simulink model "SeatClimaPreProcMgr"  (R2022b)
settings: Solver=FixedStepDiscrete  StartTime=0.0  StopTime=10.0  FixedStep=0.1  RelTol=1e-3
settings Model: Creator=blue.ge  LastModifiedBy=blue.ge
settings DataIOCC: SaveFormat=StructureWithTime
settings OptimizationCC: DefaultParameterBehavior=Inlined  EnhancedBackFolding=on  PassReuseOutputArgsAs=Individual arguments  ZeroExternalMemoryAtStartup=off  ZeroInternalMemoryAtStartup=off  OptimizeBlockOrder=speed  DifferentSizesBufferReuse=on  OptimizationCustomize=off  EfficientTunableParamExpr=on
settings DebuggingCC: ParameterTunabilityLossMsg=error
settings ModelReferenceCC: ModelReferenceNumInstancesAllowed=Single
settings SFSimCC: MATLABDynamicMemAlloc=off
settings RTWCC: Description=AUTOSAR  SystemTargetFile=autosar.tlc  GenCodeOnly=on  TemplateMakefile=ert_default_tmf
settings CodeAppCC: ForceParamTrailComments=on  IgnoreCustomStorageClasses=off  OperatorAnnotations=on  SimulinkDataObjDesc=on  SFDataObjDesc=on  InsertBlockDesc=on
settings STFCustomTargetCC: TargetLangStandard=C99 (ISO)  MultiwordLength=256  ParMdlRefBuildCompliant=on  CompOptLevelCompliant=on  IncludeMdlTerminateFcn=off  CombineOutputUpdateFcns=on  SuppressErrorStatus=on  ERTCustomFileBanners=on  SupportNonFinite=off  SupportComplex=off  SupportContinuousTime=off  SupportNonInlinedSFcns=off  RemoveResetFunc=on  MATLABClassNameForMDSCustomization=Simulink.SoftwareTarget.ERTCustomization  AutosarCompliant=on  UseToolchainInfoCompliant=on  LookupTableObjectStructAxisOrder=2,1,3,4,...  LUTObjectStructOrderEvenSpacing=Size,Table,Breakpoints  SystemTargetFile=autosar.tlc
settings ERTTargetCC: TargetLangStandard=C99 (ISO)  ConcurrentExecutionCompliant=off  IncludeMdlTerminateFcn=off  SuppressErrorStatus=on  SupportNonFinite=off  SupportComplex=off  ModelStepFunctionPrototypeControlCompliant=off  CPPClassGenCompliant=off  AutosarCompliant=on  LookupTableObjectStructAxisOrder=2,1,3,4,...  LUTObjectStructOrderEvenSpacing=Size,Table,Breakpoints  InlinedParameterPlacement=NonHierarchical  ERTCustomFileTemplate=example_file_process.tlc

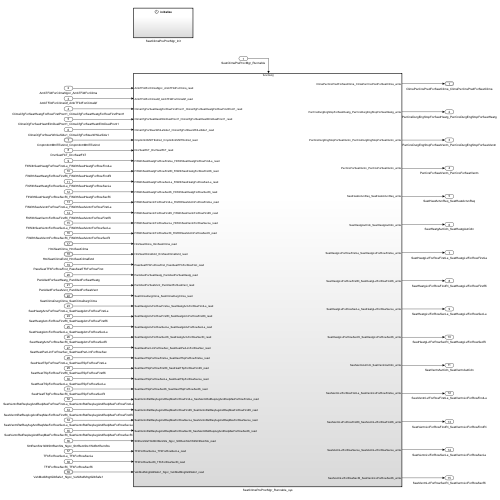
[thumbnail: Simulink's stored preview of the top-level diagram]
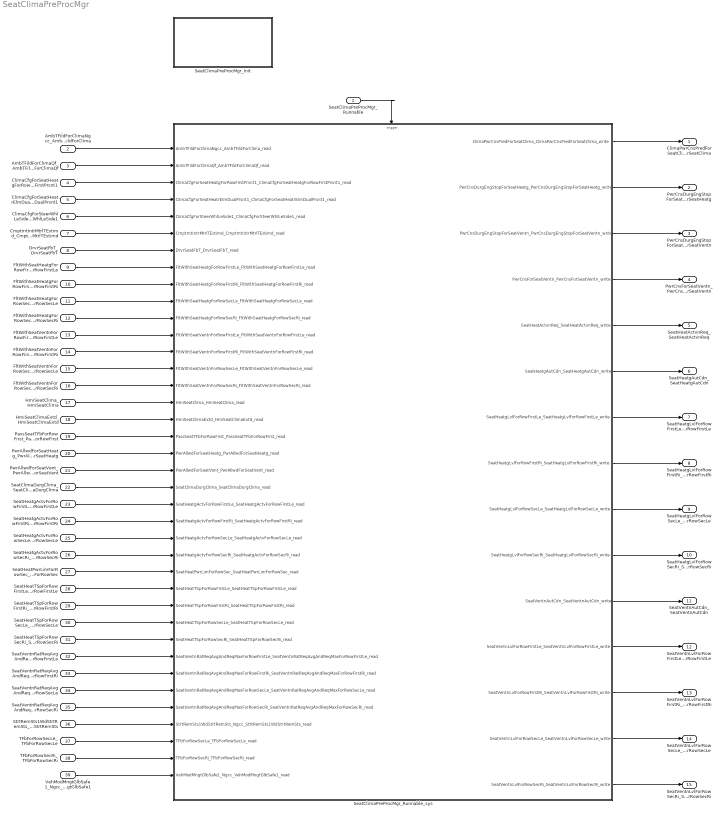
== system SeatClimaPreProcMgr ==
  SeatClimaPreProcMgr_Runnable : Inport  SampleTime=0.1  OutputFunctionCall=on
  AmbTFildForClimaNgcc_AmbTFildForClima : Inport  Port=2  OutDataTypeStr=T30  PortDimensions=1
  AmbTFildForClimaQf_AmbTFildForClimaQf : Inport  Port=3  OutDataTypeStr=Enum: GenQf1  PortDimensions=1
  ClimaCfgForSeatHeatgForRowFirstPrsnt1_ClimaCfgForSeatHeatgForRowFirstPrsnt1 : Inport  Port=4  OutDataTypeStr=Boolean  PortDimensions=1
  ClimaCfgForSeatHeatrElmDualPrsnt1_ClimaCfgForSeatHeatrElmDualPrsnt1 : Inport  Port=5  OutDataTypeStr=Boolean  PortDimensions=1
  ClimaCfgForSteerWhlLeSide1_ClimaCfgForSteerWhlLeSide1 : Inport  Port=6  OutDataTypeStr=Boolean  PortDimensions=1
  CmptmtIntrMtrlTEstimd_CmptmtIntrMtrlTEstimd : Inport  Port=7  OutDataTypeStr=Bus: MtrlSnsrT  PortDimensions=1  BusOutputAsStruct=on
  DrvrSeatFbT_DrvrSeatFbT : Inport  Port=8  OutDataTypeStr=T30  PortDimensions=1
  FltWithSeatHeatgForRowFirstLe_FltWithSeatHeatgForRowFirstLe : Inport  Port=9  OutDataTypeStr=Boolean  PortDimensions=1
  FltWithSeatHeatgForRowFirstRi_FltWithSeatHeatgForRowFirstRi : Inport  Port=10  OutDataTypeStr=Boolean  PortDimensions=1
  FltWithSeatHeatgForRowSecLe_FltWithSeatHeatgForRowSecLe : Inport  Port=11  OutDataTypeStr=Boolean  PortDimensions=1
  FltWithSeatHeatgForRowSecRi_FltWithSeatHeatgForRowSecRi : Inport  Port=12  OutDataTypeStr=Boolean  PortDimensions=1
  FltWithSeatVentnForRowFirstLe_FltWithSeatVentnForRowFirstLe : Inport  Port=13  OutDataTypeStr=Boolean  PortDimensions=1
  FltWithSeatVentnForRowFirstRi_FltWithSeatVentnForRowFirstRi : Inport  Port=14  OutDataTypeStr=Boolean  PortDimensions=1
  FltWithSeatVentnForRowSecLe_FltWithSeatVentnForRowSecLe : Inport  Port=15  OutDataTypeStr=Boolean  PortDimensions=1
  FltWithSeatVentnForRowSecRi_FltWithSeatVentnForRowSecRi : Inport  Port=16  OutDataTypeStr=Boolean  PortDimensions=1
  HmiSeatClima_HmiSeatClima : Inport  Port=17  OutDataTypeStr=Bus: HmiSeatClima  PortDimensions=1  BusOutputAsStruct=on
  HmiSeatClimaExtd_HmiSeatClimaExtd : Inport  Port=18  OutDataTypeStr=Bus: HmiSeatClimaExtd  PortDimensions=1  BusOutputAsStruct=on
  PassSeatTFbForRowFirst_PassSeatTFbForRowFirst : Inport  Port=19  OutDataTypeStr=T30  PortDimensions=1
  PwrAllwdForSeatHeatg_PwrAllwdForSeatHeatg : Inport  Port=20  OutDataTypeStr=Pwr33  PortDimensions=1
  PwrAllwdForSeatVent_PwrAllwdForSeatVent : Inport  Port=21  OutDataTypeStr=Pwr33  PortDimensions=1
  SeatClimaDurgClima_SeatClimaDurgClima : Inport  Port=22  OutDataTypeStr=Bus: HmiSeatClima  PortDimensions=1  BusOutputAsStruct=on
  SeatHeatgActvForRowFirstLe_SeatHeatgActvForRowFirstLe : Inport  Port=23  OutDataTypeStr=Boolean  PortDimensions=1
  SeatHeatgActvForRowFirstRi_SeatHeatgActvForRowFirstRi : Inport  Port=24  OutDataTypeStr=Boolean  PortDimensions=1
  SeatHeatgActvForRowSecLe_SeatHeatgActvForRowSecLe : Inport  Port=25  OutDataTypeStr=Boolean  PortDimensions=1
  SeatHeatgActvForRowSecRi_SeatHeatgActvForRowSecRi : Inport  Port=26  OutDataTypeStr=Boolean  PortDimensions=1
  SeatHeatPwrLimForRowSec_SeatHeatPwrLimForRowSec : Inport  Port=27  OutDataTypeStr=Perc30  PortDimensions=1
  SeatHeatTSpForRowFirstLe_SeatHeatTSpForRowFirstLe : Inport  Port=28  OutDataTypeStr=T30  PortDimensions=1
  SeatHeatTSpForRowFirstRi_SeatHeatTSpForRowFirstRi : Inport  Port=29  OutDataTypeStr=T30  PortDimensions=1
  SeatHeatTSpForRowSecLe_SeatHeatTSpForRowSecLe : Inport  Port=30  OutDataTypeStr=T30  PortDimensions=1
  SeatHeatTSpForRowSecRi_SeatHeatTSpForRowSecRi : Inport  Port=31  OutDataTypeStr=T30  PortDimensions=1
  SeatVentnRatReqAvgAndReqMaxForRowFirstLe_SeatVentnRatReqAvgAndReqMaxForRowFirstLe : Inport  Port=32  OutDataTypeStr=Fac30  PortDimensions=1
  SeatVentnRatReqAvgAndReqMaxForRowFirstRi_SeatVentnRatReqAvgAndReqMaxForRowFirstRi : Inport  Port=33  OutDataTypeStr=Fac30  PortDimensions=1
  SeatVentnRatReqAvgAndReqMaxForRowSecLe_SeatVentnRatReqAvgAndReqMaxForRowSecLe : Inport  Port=34  OutDataTypeStr=Fac30  PortDimensions=1
  SeatVentnRatReqAvgAndReqMaxForRowSecRi_SeatVentnRatReqAvgAndReqMaxForRowSecRi : Inport  Port=35  OutDataTypeStr=Fac30  PortDimensions=1
  StrtRemSts1WdStrtRemSts_Ngcc_StrtRemSts1WdStrtRemSts : Inport  Port=36  OutDataTypeStr=Enum: StrtRemSts1  PortDimensions=1
  TFbForRowSecLe_TFbForRowSecLe : Inport  Port=37  OutDataTypeStr=T30  PortDimensions=1
  TFbForRowSecRi_TFbForRowSecRi : Inport  Port=38  OutDataTypeStr=T30  PortDimensions=1
  VehModMngtGlbSafe1_Ngcc_VehModMngtGlbSafe1 : Inport  Port=39  OutDataTypeStr=Bus: VehModMngtGlbSafe1  PortDimensions=1  BusOutputAsStruct=on
  SeatClimaPreProcMgr_Init : Subsystem
  SeatClimaPreProcMgr_Runnable_sys : Subsystem
  ClimaPwrCnsPredForSeatClima_ClimaPwrCnsPredForSeatClima : Outport  OutDataTypeStr=Bus: ClimaPwrCnsPredRec1  PortDimensions=1  BusOutputAsStruct=on
  PwrCnsDurgEngStopForSeatHeatg_PwrCnsDurgEngStopForSeatHeatg : Outport  Port=2  OutDataTypeStr=Pwr33  PortDimensions=1
  PwrCnsDurgEngStopForSeatVentn_PwrCnsDurgEngStopForSeatVentn : Outport  Port=3  OutDataTypeStr=Pwr33  PortDimensions=1
  PwrCnsForSeatVentn_PwrCnsForSeatVentn : Outport  Port=4  OutDataTypeStr=Pwr33  PortDimensions=1
  SeatHeatActvnReq_SeatHeatActvnReq : Outport  Port=5  OutDataTypeStr=Enum: OnOff1  PortDimensions=1
  SeatHeatgAutCdn_SeatHeatgAutCdn : Outport  Port=6  OutDataTypeStr=Enum: Flg1  PortDimensions=1
  SeatHeatgLvlForRowFirstLe_SeatHeatgLvlForRowFirstLe : Outport  Port=7  OutDataTypeStr=Enum: SeatClimaLvl  PortDimensions=1
  SeatHeatgLvlForRowFirstRi_SeatHeatgLvlForRowFirstRi : Outport  Port=8  OutDataTypeStr=Enum: SeatClimaLvl  PortDimensions=1
  SeatHeatgLvlForRowSecLe_SeatHeatgLvlForRowSecLe : Outport  Port=9  OutDataTypeStr=Enum: SeatClimaLvl  PortDimensions=1
  SeatHeatgLvlForRowSecRi_SeatHeatgLvlForRowSecRi : Outport  Port=10  OutDataTypeStr=Enum: SeatClimaLvl  PortDimensions=1
  SeatVentnAutCdn_SeatVentnAutCdn : Outport  Port=11  OutDataTypeStr=Enum: Flg1  PortDimensions=1
  SeatVentnLvlForRowFirstLe_SeatVentnLvlForRowFirstLe : Outport  Port=12  OutDataTypeStr=Enum: SeatClimaLvl  PortDimensions=1
  SeatVentnLvlForRowFirstRi_SeatVentnLvlForRowFirstRi : Outport  Port=13  OutDataTypeStr=Enum: SeatClimaLvl  PortDimensions=1
  SeatVentnLvlForRowSecLe_SeatVentnLvlForRowSecLe : Outport  Port=14  OutDataTypeStr=Enum: SeatClimaLvl  PortDimensions=1
  SeatVentnLvlForRowSecRi_SeatVentnLvlForRowSecRi : Outport  Port=15  OutDataTypeStr=Enum: SeatClimaLvl  PortDimensions=1
  wires:
  AmbTFildForClimaNgcc_AmbTFildForClima/1 -> SeatClimaPreProcMgr_Runnable_sys/1(AmbTFildForClimaNgcc_AmbTFildForClima_read)
  AmbTFildForClimaQf_AmbTFildForClimaQf/1 -> SeatClimaPreProcMgr_Runnable_sys/2(AmbTFildForClimaQf_AmbTFildForClimaQf_read)
  ClimaCfgForSeatHeatgForRowFirstPrsnt1_ClimaCfgForSeatHeatgForRowFirstPrsnt1/1 -> SeatClimaPreProcMgr_Runnable_sys/3(ClimaCfgForSeatHeatgForRowFirstPrsnt1_ClimaCfgForSeatHeatgForRowFirstPrsnt1_read)
  ClimaCfgForSeatHeatrElmDualPrsnt1_ClimaCfgForSeatHeatrElmDualPrsnt1/1 -> SeatClimaPreProcMgr_Runnable_sys/4(ClimaCfgForSeatHeatrElmDualPrsnt1_ClimaCfgForSeatHeatrElmDualPrsnt1_read)
  ClimaCfgForSteerWhlLeSide1_ClimaCfgForSteerWhlLeSide1/1 -> SeatClimaPreProcMgr_Runnable_sys/5(ClimaCfgForSteerWhlLeSide1_ClimaCfgForSteerWhlLeSide1_read)
  CmptmtIntrMtrlTEstimd_CmptmtIntrMtrlTEstimd/1 -> SeatClimaPreProcMgr_Runnable_sys/6(CmptmtIntrMtrlTEstimd_CmptmtIntrMtrlTEstimd_read)
  DrvrSeatFbT_DrvrSeatFbT/1 -> SeatClimaPreProcMgr_Runnable_sys/7(DrvrSeatFbT_DrvrSeatFbT_read)
  FltWithSeatHeatgForRowFirstLe_FltWithSeatHeatgForRowFirstLe/1 -> SeatClimaPreProcMgr_Runnable_sys/8(FltWithSeatHeatgForRowFirstLe_FltWithSeatHeatgForRowFirstLe_read)
  FltWithSeatHeatgForRowFirstRi_FltWithSeatHeatgForRowFirstRi/1 -> SeatClimaPreProcMgr_Runnable_sys/9(FltWithSeatHeatgForRowFirstRi_FltWithSeatHeatgForRowFirstRi_read)
  FltWithSeatHeatgForRowSecLe_FltWithSeatHeatgForRowSecLe/1 -> SeatClimaPreProcMgr_Runnable_sys/10(FltWithSeatHeatgForRowSecLe_FltWithSeatHeatgForRowSecLe_read)
  FltWithSeatHeatgForRowSecRi_FltWithSeatHeatgForRowSecRi/1 -> SeatClimaPreProcMgr_Runnable_sys/11(FltWithSeatHeatgForRowSecRi_FltWithSeatHeatgForRowSecRi_read)
  FltWithSeatVentnForRowFirstLe_FltWithSeatVentnForRowFirstLe/1 -> SeatClimaPreProcMgr_Runnable_sys/12(FltWithSeatVentnForRowFirstLe_FltWithSeatVentnForRowFirstLe_read)
  FltWithSeatVentnForRowFirstRi_FltWithSeatVentnForRowFirstRi/1 -> SeatClimaPreProcMgr_Runnable_sys/13(FltWithSeatVentnForRowFirstRi_FltWithSeatVentnForRowFirstRi_read)
  FltWithSeatVentnForRowSecLe_FltWithSeatVentnForRowSecLe/1 -> SeatClimaPreProcMgr_Runnable_sys/14(FltWithSeatVentnForRowSecLe_FltWithSeatVentnForRowSecLe_read)
  FltWithSeatVentnForRowSecRi_FltWithSeatVentnForRowSecRi/1 -> SeatClimaPreProcMgr_Runnable_sys/15(FltWithSeatVentnForRowSecRi_FltWithSeatVentnForRowSecRi_read)
  HmiSeatClima_HmiSeatClima/1 -> SeatClimaPreProcMgr_Runnable_sys/16(HmiSeatClima_HmiSeatClima_read)
  HmiSeatClimaExtd_HmiSeatClimaExtd/1 -> SeatClimaPreProcMgr_Runnable_sys/17(HmiSeatClimaExtd_HmiSeatClimaExtd_read)
  PassSeatTFbForRowFirst_PassSeatTFbForRowFirst/1 -> SeatClimaPreProcMgr_Runnable_sys/18(PassSeatTFbForRowFirst_PassSeatTFbForRowFirst_read)
  PwrAllwdForSeatHeatg_PwrAllwdForSeatHeatg/1 -> SeatClimaPreProcMgr_Runnable_sys/19(PwrAllwdForSeatHeatg_PwrAllwdForSeatHeatg_read)
  PwrAllwdForSeatVent_PwrAllwdForSeatVent/1 -> SeatClimaPreProcMgr_Runnable_sys/20(PwrAllwdForSeatVent_PwrAllwdForSeatVent_read)
  SeatClimaDurgClima_SeatClimaDurgClima/1 -> SeatClimaPreProcMgr_Runnable_sys/21(SeatClimaDurgClima_SeatClimaDurgClima_read)
  SeatHeatgActvForRowFirstLe_SeatHeatgActvForRowFirstLe/1 -> SeatClimaPreProcMgr_Runnable_sys/22(SeatHeatgActvForRowFirstLe_SeatHeatgActvForRowFirstLe_read)
  SeatHeatgActvForRowFirstRi_SeatHeatgActvForRowFirstRi/1 -> SeatClimaPreProcMgr_Runnable_sys/23(SeatHeatgActvForRowFirstRi_SeatHeatgActvForRowFirstRi_read)
  SeatHeatgActvForRowSecLe_SeatHeatgActvForRowSecLe/1 -> SeatClimaPreProcMgr_Runnable_sys/24(SeatHeatgActvForRowSecLe_SeatHeatgActvForRowSecLe_read)
  SeatHeatgActvForRowSecRi_SeatHeatgActvForRowSecRi/1 -> SeatClimaPreProcMgr_Runnable_sys/25(SeatHeatgActvForRowSecRi_SeatHeatgActvForRowSecRi_read)
  SeatHeatPwrLimForRowSec_SeatHeatPwrLimForRowSec/1 -> SeatClimaPreProcMgr_Runnable_sys/26(SeatHeatPwrLimForRowSec_SeatHeatPwrLimForRowSec_read)
  SeatHeatTSpForRowFirstLe_SeatHeatTSpForRowFirstLe/1 -> SeatClimaPreProcMgr_Runnable_sys/27(SeatHeatTSpForRowFirstLe_SeatHeatTSpForRowFirstLe_read)
  SeatHeatTSpForRowFirstRi_SeatHeatTSpForRowFirstRi/1 -> SeatClimaPreProcMgr_Runnable_sys/28(SeatHeatTSpForRowFirstRi_SeatHeatTSpForRowFirstRi_read)
  SeatHeatTSpForRowSecLe_SeatHeatTSpForRowSecLe/1 -> SeatClimaPreProcMgr_Runnable_sys/29(SeatHeatTSpForRowSecLe_SeatHeatTSpForRowSecLe_read)
  SeatHeatTSpForRowSecRi_SeatHeatTSpForRowSecRi/1 -> SeatClimaPreProcMgr_Runnable_sys/30(SeatHeatTSpForRowSecRi_SeatHeatTSpForRowSecRi_read)
  SeatVentnRatReqAvgAndReqMaxForRowFirstLe_SeatVentnRatReqAvgAndReqMaxForRowFirstLe/1 -> SeatClimaPreProcMgr_Runnable_sys/31(SeatVentnRatReqAvgAndReqMaxForRowFirstLe_SeatVentnRatReqAvgAndReqMaxForRowFirstLe_read)
  SeatVentnRatReqAvgAndReqMaxForRowFirstRi_SeatVentnRatReqAvgAndReqMaxForRowFirstRi/1 -> SeatClimaPreProcMgr_Runnable_sys/32(SeatVentnRatReqAvgAndReqMaxForRowFirstRi_SeatVentnRatReqAvgAndReqMaxForRowFirstRi_read)
  SeatVentnRatReqAvgAndReqMaxForRowSecLe_SeatVentnRatReqAvgAndReqMaxForRowSecLe/1 -> SeatClimaPreProcMgr_Runnable_sys/33(SeatVentnRatReqAvgAndReqMaxForRowSecLe_SeatVentnRatReqAvgAndReqMaxForRowSecLe_read)
  SeatVentnRatReqAvgAndReqMaxForRowSecRi_SeatVentnRatReqAvgAndReqMaxForRowSecRi/1 -> SeatClimaPreProcMgr_Runnable_sys/34(SeatVentnRatReqAvgAndReqMaxForRowSecRi_SeatVentnRatReqAvgAndReqMaxForRowSecRi_read)
  StrtRemSts1WdStrtRemSts_Ngcc_StrtRemSts1WdStrtRemSts/1 -> SeatClimaPreProcMgr_Runnable_sys/35(StrtRemSts1WdStrtRemSts_Ngcc_StrtRemSts1WdStrtRemSts_read)
  TFbForRowSecLe_TFbForRowSecLe/1 -> SeatClimaPreProcMgr_Runnable_sys/36(TFbForRowSecLe_TFbForRowSecLe_read)
  TFbForRowSecRi_TFbForRowSecRi/1 -> SeatClimaPreProcMgr_Runnable_sys/37(TFbForRowSecRi_TFbForRowSecRi_read)
  VehModMngtGlbSafe1_Ngcc_VehModMngtGlbSafe1/1 -> SeatClimaPreProcMgr_Runnable_sys/38(VehModMngtGlbSafe1_Ngcc_VehModMngtGlbSafe1_read)
  SeatClimaPreProcMgr_Runnable_sys/1(ClimaPwrCnsPredForSeatClima_ClimaPwrCnsPredForSeatClima_write) -> ClimaPwrCnsPredForSeatClima_ClimaPwrCnsPredForSeatClima/1
  SeatClimaPreProcMgr_Runnable_sys/2(PwrCnsDurgEngStopForSeatHeatg_PwrCnsDurgEngStopForSeatHeatg_write) -> PwrCnsDurgEngStopForSeatHeatg_PwrCnsDurgEngStopForSeatHeatg/1
  SeatClimaPreProcMgr_Runnable_sys/3(PwrCnsDurgEngStopForSeatVentn_PwrCnsDurgEngStopForSeatVentn_write) -> PwrCnsDurgEngStopForSeatVentn_PwrCnsDurgEngStopForSeatVentn/1
  SeatClimaPreProcMgr_Runnable_sys/4(PwrCnsForSeatVentn_PwrCnsForSeatVentn_write) -> PwrCnsForSeatVentn_PwrCnsForSeatVentn/1
  SeatClimaPreProcMgr_Runnable_sys/5(SeatHeatActvnReq_SeatHeatActvnReq_write) -> SeatHeatActvnReq_SeatHeatActvnReq/1
  SeatClimaPreProcMgr_Runnable_sys/6(SeatHeatgAutCdn_SeatHeatgAutCdn_write) -> SeatHeatgAutCdn_SeatHeatgAutCdn/1
  SeatClimaPreProcMgr_Runnable_sys/7(SeatHeatgLvlForRowFirstLe_SeatHeatgLvlForRowFirstLe_write) -> SeatHeatgLvlForRowFirstLe_SeatHeatgLvlForRowFirstLe/1
  SeatClimaPreProcMgr_Runnable_sys/8(SeatHeatgLvlForRowFirstRi_SeatHeatgLvlForRowFirstRi_write) -> SeatHeatgLvlForRowFirstRi_SeatHeatgLvlForRowFirstRi/1
  SeatClimaPreProcMgr_Runnable_sys/9(SeatHeatgLvlForRowSecLe_SeatHeatgLvlForRowSecLe_write) -> SeatHeatgLvlForRowSecLe_SeatHeatgLvlForRowSecLe/1
  SeatClimaPreProcMgr_Runnable_sys/10(SeatHeatgLvlForRowSecRi_SeatHeatgLvlForRowSecRi_write) -> SeatHeatgLvlForRowSecRi_SeatHeatgLvlForRowSecRi/1
  SeatClimaPreProcMgr_Runnable_sys/11(SeatVentnAutCdn_SeatVentnAutCdn_write) -> SeatVentnAutCdn_SeatVentnAutCdn/1
  SeatClimaPreProcMgr_Runnable_sys/12(SeatVentnLvlForRowFirstLe_SeatVentnLvlForRowFirstLe_write) -> SeatVentnLvlForRowFirstLe_SeatVentnLvlForRowFirstLe/1
  SeatClimaPreProcMgr_Runnable_sys/13(SeatVentnLvlForRowFirstRi_SeatVentnLvlForRowFirstRi_write) -> SeatVentnLvlForRowFirstRi_SeatVentnLvlForRowFirstRi/1
  SeatClimaPreProcMgr_Runnable_sys/14(SeatVentnLvlForRowSecLe_SeatVentnLvlForRowSecLe_write) -> SeatVentnLvlForRowSecLe_SeatVentnLvlForRowSecLe/1
  SeatClimaPreProcMgr_Runnable_sys/15(SeatVentnLvlForRowSecRi_SeatVentnLvlForRowSecRi_write) -> SeatVentnLvlForRowSecRi_SeatVentnLvlForRowSecRi/1
  SeatClimaPreProcMgr_Runnable/1 -> SeatClimaPreProcMgr_Runnable_sys/Trigger

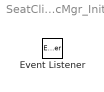
== system SeatClimaPreProcMgr/SeatClimaPreProcMgr_Init ==
  Event Listener : EventListener  EventName=reset

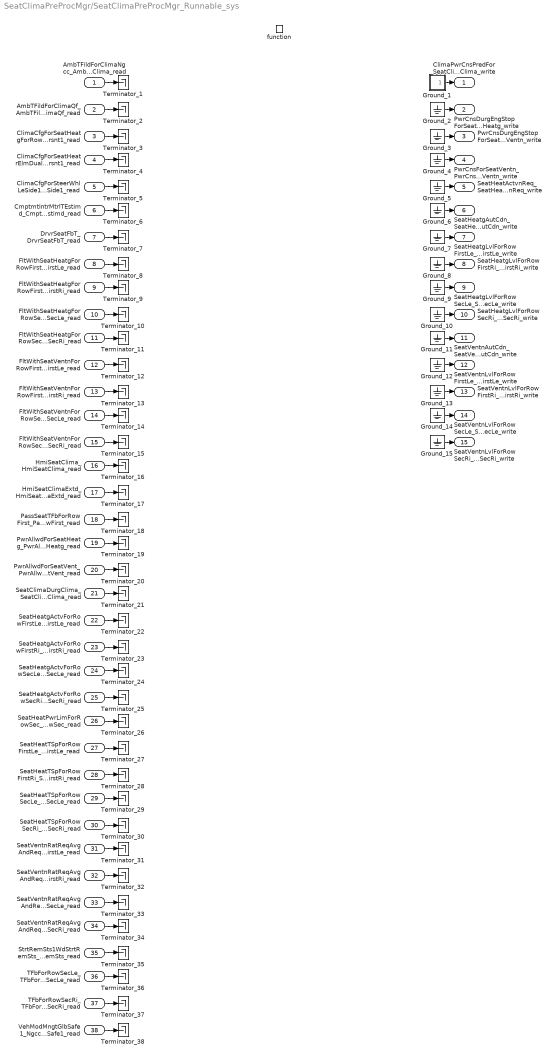
== system SeatClimaPreProcMgr/SeatClimaPreProcMgr_Runnable_sys ==
  AmbTFildForClimaNgcc_AmbTFildForClima_read : Inport
  AmbTFildForClimaQf_AmbTFildForClimaQf_read : Inport  Port=2
  ClimaCfgForSeatHeatgForRowFirstPrsnt1_ClimaCfgForSeatHeatgForRowFirstPrsnt1_read : Inport  Port=3
  ClimaCfgForSeatHeatrElmDualPrsnt1_ClimaCfgForSeatHeatrElmDualPrsnt1_read : Inport  Port=4
  ClimaCfgForSteerWhlLeSide1_ClimaCfgForSteerWhlLeSide1_read : Inport  Port=5
  CmptmtIntrMtrlTEstimd_CmptmtIntrMtrlTEstimd_read : Inport  Port=6
  DrvrSeatFbT_DrvrSeatFbT_read : Inport  Port=7
  FltWithSeatHeatgForRowFirstLe_FltWithSeatHeatgForRowFirstLe_read : Inport  Port=8
  FltWithSeatHeatgForRowFirstRi_FltWithSeatHeatgForRowFirstRi_read : Inport  Port=9
  FltWithSeatHeatgForRowSecLe_FltWithSeatHeatgForRowSecLe_read : Inport  Port=10
  FltWithSeatHeatgForRowSecRi_FltWithSeatHeatgForRowSecRi_read : Inport  Port=11
  FltWithSeatVentnForRowFirstLe_FltWithSeatVentnForRowFirstLe_read : Inport  Port=12
  FltWithSeatVentnForRowFirstRi_FltWithSeatVentnForRowFirstRi_read : Inport  Port=13
  FltWithSeatVentnForRowSecLe_FltWithSeatVentnForRowSecLe_read : Inport  Port=14
  FltWithSeatVentnForRowSecRi_FltWithSeatVentnForRowSecRi_read : Inport  Port=15
  HmiSeatClima_HmiSeatClima_read : Inport  Port=16
  HmiSeatClimaExtd_HmiSeatClimaExtd_read : Inport  Port=17
  PassSeatTFbForRowFirst_PassSeatTFbForRowFirst_read : Inport  Port=18
  PwrAllwdForSeatHeatg_PwrAllwdForSeatHeatg_read : Inport  Port=19
  PwrAllwdForSeatVent_PwrAllwdForSeatVent_read : Inport  Port=20
  SeatClimaDurgClima_SeatClimaDurgClima_read : Inport  Port=21
  SeatHeatgActvForRowFirstLe_SeatHeatgActvForRowFirstLe_read : Inport  Port=22
  SeatHeatgActvForRowFirstRi_SeatHeatgActvForRowFirstRi_read : Inport  Port=23
  SeatHeatgActvForRowSecLe_SeatHeatgActvForRowSecLe_read : Inport  Port=24
  SeatHeatgActvForRowSecRi_SeatHeatgActvForRowSecRi_read : Inport  Port=25
  SeatHeatPwrLimForRowSec_SeatHeatPwrLimForRowSec_read : Inport  Port=26
  SeatHeatTSpForRowFirstLe_SeatHeatTSpForRowFirstLe_read : Inport  Port=27
  SeatHeatTSpForRowFirstRi_SeatHeatTSpForRowFirstRi_read : Inport  Port=28
  SeatHeatTSpForRowSecLe_SeatHeatTSpForRowSecLe_read : Inport  Port=29
  SeatHeatTSpForRowSecRi_SeatHeatTSpForRowSecRi_read : Inport  Port=30
  SeatVentnRatReqAvgAndReqMaxForRowFirstLe_SeatVentnRatReqAvgAndReqMaxForRowFirstLe_read : Inport  Port=31
  SeatVentnRatReqAvgAndReqMaxForRowFirstRi_SeatVentnRatReqAvgAndReqMaxForRowFirstRi_read : Inport  Port=32
  SeatVentnRatReqAvgAndReqMaxForRowSecLe_SeatVentnRatReqAvgAndReqMaxForRowSecLe_read : Inport  Port=33
  SeatVentnRatReqAvgAndReqMaxForRowSecRi_SeatVentnRatReqAvgAndReqMaxForRowSecRi_read : Inport  Port=34
  StrtRemSts1WdStrtRemSts_Ngcc_StrtRemSts1WdStrtRemSts_read : Inport  Port=35
  TFbForRowSecLe_TFbForRowSecLe_read : Inport  Port=36
  TFbForRowSecRi_TFbForRowSecRi_read : Inport  Port=37
  VehModMngtGlbSafe1_Ngcc_VehModMngtGlbSafe1_read : Inport  Port=38
  function : TriggerPort  TriggerType=function-call  VariantControl=(inherit)  SampleTimeType=periodic  SampleTime=-1
  Ground_1 : Subsystem
  Ground_{2..15} (14 alike) : Ground
  Terminator_{1..38} (38 alike) : Terminator
  ClimaPwrCnsPredForSeatClima_ClimaPwrCnsPredForSeatClima_write : Outport
      settings: EnsureOutportIsVirtual=on
  PwrCnsDurgEngStopForSeatHeatg_PwrCnsDurgEngStopForSeatHeatg_write : Outport  Port=2
      settings: EnsureOutportIsVirtual=on
  PwrCnsDurgEngStopForSeatVentn_PwrCnsDurgEngStopForSeatVentn_write : Outport  Port=3
      settings: EnsureOutportIsVirtual=on
  PwrCnsForSeatVentn_PwrCnsForSeatVentn_write : Outport  Port=4
      settings: EnsureOutportIsVirtual=on
  SeatHeatActvnReq_SeatHeatActvnReq_write : Outport  Port=5
      settings: EnsureOutportIsVirtual=on
  SeatHeatgAutCdn_SeatHeatgAutCdn_write : Outport  Port=6
      settings: EnsureOutportIsVirtual=on
  SeatHeatgLvlForRowFirstLe_SeatHeatgLvlForRowFirstLe_write : Outport  Port=7
      settings: EnsureOutportIsVirtual=on
  SeatHeatgLvlForRowFirstRi_SeatHeatgLvlForRowFirstRi_write : Outport  Port=8
      settings: EnsureOutportIsVirtual=on
  SeatHeatgLvlForRowSecLe_SeatHeatgLvlForRowSecLe_write : Outport  Port=9
      settings: EnsureOutportIsVirtual=on
  SeatHeatgLvlForRowSecRi_SeatHeatgLvlForRowSecRi_write : Outport  Port=10
      settings: EnsureOutportIsVirtual=on
  SeatVentnAutCdn_SeatVentnAutCdn_write : Outport  Port=11
      settings: EnsureOutportIsVirtual=on
  SeatVentnLvlForRowFirstLe_SeatVentnLvlForRowFirstLe_write : Outport  Port=12
      settings: EnsureOutportIsVirtual=on
  SeatVentnLvlForRowFirstRi_SeatVentnLvlForRowFirstRi_write : Outport  Port=13
      settings: EnsureOutportIsVirtual=on
  SeatVentnLvlForRowSecLe_SeatVentnLvlForRowSecLe_write : Outport  Port=14
      settings: EnsureOutportIsVirtual=on
  SeatVentnLvlForRowSecRi_SeatVentnLvlForRowSecRi_write : Outport  Port=15
      settings: EnsureOutportIsVirtual=on
  wires:
  AmbTFildForClimaNgcc_AmbTFildForClima_read/1 -> Terminator_1/1
  AmbTFildForClimaQf_AmbTFildForClimaQf_read/1 -> Terminator_2/1
  ClimaCfgForSeatHeatgForRowFirstPrsnt1_ClimaCfgForSeatHeatgForRowFirstPrsnt1_read/1 -> Terminator_3/1
  ClimaCfgForSeatHeatrElmDualPrsnt1_ClimaCfgForSeatHeatrElmDualPrsnt1_read/1 -> Terminator_4/1
  ClimaCfgForSteerWhlLeSide1_ClimaCfgForSteerWhlLeSide1_read/1 -> Terminator_5/1
  CmptmtIntrMtrlTEstimd_CmptmtIntrMtrlTEstimd_read/1 -> Terminator_6/1
  DrvrSeatFbT_DrvrSeatFbT_read/1 -> Terminator_7/1
  FltWithSeatHeatgForRowFirstLe_FltWithSeatHeatgForRowFirstLe_read/1 -> Terminator_8/1
  FltWithSeatHeatgForRowFirstRi_FltWithSeatHeatgForRowFirstRi_read/1 -> Terminator_9/1
  FltWithSeatHeatgForRowSecLe_FltWithSeatHeatgForRowSecLe_read/1 -> Terminator_10/1
  FltWithSeatHeatgForRowSecRi_FltWithSeatHeatgForRowSecRi_read/1 -> Terminator_11/1
  FltWithSeatVentnForRowFirstLe_FltWithSeatVentnForRowFirstLe_read/1 -> Terminator_12/1
  FltWithSeatVentnForRowFirstRi_FltWithSeatVentnForRowFirstRi_read/1 -> Terminator_13/1
  FltWithSeatVentnForRowSecLe_FltWithSeatVentnForRowSecLe_read/1 -> Terminator_14/1
  FltWithSeatVentnForRowSecRi_FltWithSeatVentnForRowSecRi_read/1 -> Terminator_15/1
  HmiSeatClima_HmiSeatClima_read/1 -> Terminator_16/1
  HmiSeatClimaExtd_HmiSeatClimaExtd_read/1 -> Terminator_17/1
  PassSeatTFbForRowFirst_PassSeatTFbForRowFirst_read/1 -> Terminator_18/1
  PwrAllwdForSeatHeatg_PwrAllwdForSeatHeatg_read/1 -> Terminator_19/1
  PwrAllwdForSeatVent_PwrAllwdForSeatVent_read/1 -> Terminator_20/1
  SeatClimaDurgClima_SeatClimaDurgClima_read/1 -> Terminator_21/1
  SeatHeatgActvForRowFirstLe_SeatHeatgActvForRowFirstLe_read/1 -> Terminator_22/1
  SeatHeatgActvForRowFirstRi_SeatHeatgActvForRowFirstRi_read/1 -> Terminator_23/1
  SeatHeatgActvForRowSecLe_SeatHeatgActvForRowSecLe_read/1 -> Terminator_24/1
  SeatHeatgActvForRowSecRi_SeatHeatgActvForRowSecRi_read/1 -> Terminator_25/1
  SeatHeatPwrLimForRowSec_SeatHeatPwrLimForRowSec_read/1 -> Terminator_26/1
  SeatHeatTSpForRowFirstLe_SeatHeatTSpForRowFirstLe_read/1 -> Terminator_27/1
  SeatHeatTSpForRowFirstRi_SeatHeatTSpForRowFirstRi_read/1 -> Terminator_28/1
  SeatHeatTSpForRowSecLe_SeatHeatTSpForRowSecLe_read/1 -> Terminator_29/1
  SeatHeatTSpForRowSecRi_SeatHeatTSpForRowSecRi_read/1 -> Terminator_30/1
  SeatVentnRatReqAvgAndReqMaxForRowFirstLe_SeatVentnRatReqAvgAndReqMaxForRowFirstLe_read/1 -> Terminator_31/1
  SeatVentnRatReqAvgAndReqMaxForRowFirstRi_SeatVentnRatReqAvgAndReqMaxForRowFirstRi_read/1 -> Terminator_32/1
  SeatVentnRatReqAvgAndReqMaxForRowSecLe_SeatVentnRatReqAvgAndReqMaxForRowSecLe_read/1 -> Terminator_33/1
  SeatVentnRatReqAvgAndReqMaxForRowSecRi_SeatVentnRatReqAvgAndReqMaxForRowSecRi_read/1 -> Terminator_34/1
  StrtRemSts1WdStrtRemSts_Ngcc_StrtRemSts1WdStrtRemSts_read/1 -> Terminator_35/1
  TFbForRowSecLe_TFbForRowSecLe_read/1 -> Terminator_36/1
  TFbForRowSecRi_TFbForRowSecRi_read/1 -> Terminator_37/1
  VehModMngtGlbSafe1_Ngcc_VehModMngtGlbSafe1_read/1 -> Terminator_38/1
  Ground_1/1(Out1) -> ClimaPwrCnsPredForSeatClima_ClimaPwrCnsPredForSeatClima_write/1
  Ground_2/1 -> PwrCnsDurgEngStopForSeatHeatg_PwrCnsDurgEngStopForSeatHeatg_write/1
  Ground_3/1 -> PwrCnsDurgEngStopForSeatVentn_PwrCnsDurgEngStopForSeatVentn_write/1
  Ground_4/1 -> PwrCnsForSeatVentn_PwrCnsForSeatVentn_write/1
  Ground_5/1 -> SeatHeatActvnReq_SeatHeatActvnReq_write/1
  Ground_6/1 -> SeatHeatgAutCdn_SeatHeatgAutCdn_write/1
  Ground_7/1 -> SeatHeatgLvlForRowFirstLe_SeatHeatgLvlForRowFirstLe_write/1
  Ground_8/1 -> SeatHeatgLvlForRowFirstRi_SeatHeatgLvlForRowFirstRi_write/1
  Ground_9/1 -> SeatHeatgLvlForRowSecLe_SeatHeatgLvlForRowSecLe_write/1
  Ground_10/1 -> SeatHeatgLvlForRowSecRi_SeatHeatgLvlForRowSecRi_write/1
  Ground_11/1 -> SeatVentnAutCdn_SeatVentnAutCdn_write/1
  Ground_12/1 -> SeatVentnLvlForRowFirstLe_SeatVentnLvlForRowFirstLe_write/1
  Ground_13/1 -> SeatVentnLvlForRowFirstRi_SeatVentnLvlForRowFirstRi_write/1
  Ground_14/1 -> SeatVentnLvlForRowSecLe_SeatVentnLvlForRowSecLe_write/1
  Ground_15/1 -> SeatVentnLvlForRowSecRi_SeatVentnLvlForRowSecRi_write/1

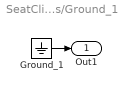
== system SeatClimaPreProcMgr/SeatClimaPreProcMgr_Runnable_sys/Ground_1 ==
  Ground_1 : Ground
  Out1 : Outport  OutDataTypeStr=Bus: ClimaPwrCnsPredRec1  PortDimensions=1
  wires:
  Ground_1/1 -> Out1/1
